simulink model "Performance_analyzer_system_design "  (R2024b)
settings: Solver=VariableStepAuto  StartTime=0.0  StopTime=10.0  RelTol=1e-3
model workspace (MAT data in the model file):
  armature_current = Simulink.Bus
  armature_current.Alignment = -1
  armature_current.PreserveElementDimensions = 0
  armature_current.DataScope = 'Auto'
  line_current = Simulink.Bus
  line_current.Alignment = -1
  line_current.PreserveElementDimensions = 0
  line_current.DataScope = 'Auto'
  speed = Simulink.Bus
  speed.Alignment = -1
  speed.PreserveElementDimensions = 0
  speed.DataScope = 'Auto'
  voltage = Simulink.Bus
  voltage.Alignment = -1
  voltage.PreserveElementDimensions = 0
  voltage.DataScope = 'Auto'

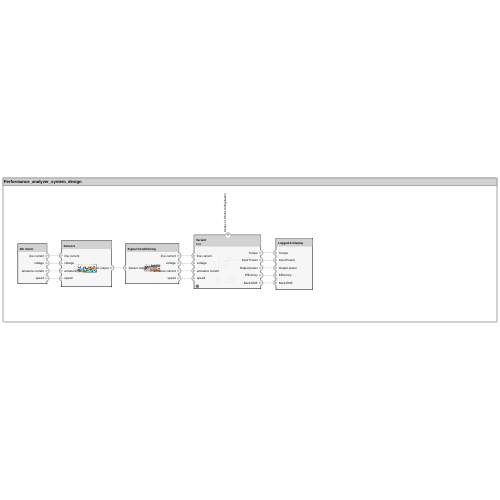
[thumbnail: Simulink's stored preview of the top-level diagram]
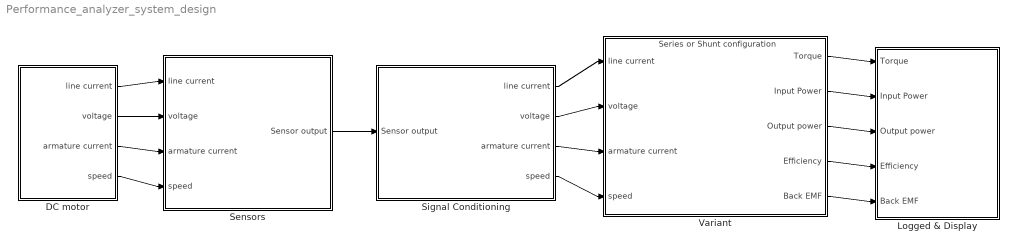
== system Performance_analyzer_system_design  ==
  "DC motor " : Subsystem
      settings: PortSchema="{\"entries\":[{\"content\":{\"headerSize\":26,\"margin\":15,\"minimumSpacing\":25,\"sides\":[{\"content\":{},\"type\":\"ConnectorPlacement.EquallySpacedRectSide\",\"uuid\":\"fe77f070-63ce-405b-ab97-62bbe056a185\"},{\"content\":{\"connectorIds\":[\"Out1\",\"Out2\",\"Out3\",\"Out4\"],\"side\":\"RIGHT\"},\"type\":\"ConnectorPlacement.EquallySpacedRectSide\",\"uuid\":\"342bb455-6b0a-4e5a-b866-05066d23c94e\"},{\"content\":{\"side\":\"TOP\"},\"type\":\"ConnectorPlacement.EquallySpacedRectSide\",\"uuid\":\"f5606689-d5e5-4d9b-a00c-e5773c57f91e\"}]},\"type\":\"ConnectorPlacement.EquallySpacedConnector\",\"uuid\":\"96da3435-ef87-4569-ba54-24bba7686016\"}],\"packageUris\":[\"http://schema.mathworks.com/mf0/Connector/R2020b\"],\"version\":\"1.0\"}"
  Logged & Display : Subsystem
      settings: PortSchema="{\"entries\":[{\"content\":{\"headerSize\":26,\"margin\":15,\"minimumSpacing\":25,\"sides\":[{\"content\":{\"connectorIds\":[\"In1\",\"In2\",\"In3\",\"In4\",\"In5\"]},\"type\":\"ConnectorPlacement.EquallySpacedRectSide\",\"uuid\":\"fe77f070-63ce-405b-ab97-62bbe056a185\"},{\"content\":{\"side\":\"RIGHT\"},\"type\":\"ConnectorPlacement.EquallySpacedRectSide\",\"uuid\":\"342bb455-6b0a-4e5a-b866-05066d23c94e\"},{\"content\":{\"side\":\"TOP\"},\"type\":\"ConnectorPlacement.EquallySpacedRectSide\",\"uuid\":\"f5606689-d5e5-4d9b-a00c-e5773c57f91e\"}]},\"type\":\"ConnectorPlacement.EquallySpacedConnector\",\"uuid\":\"96da3435-ef87-4569-ba54-24bba7686016\"}],\"packageUris\":[\"http://schema.mathworks.com/mf0/Connector/R2020b\"],\"version\":\"1.0\"}"
  Sensors : Subsystem
      settings: PortSchema="{\"entries\":[{\"content\":{\"headerSize\":26,\"margin\":15,\"minimumSpacing\":25,\"sides\":[{\"content\":{\"connectorIds\":[\"In1\",\"In2\",\"In4\",\"In3\"]},\"type\":\"ConnectorPlacement.EquallySpacedRectSide\",\"uuid\":\"fe77f070-63ce-405b-ab97-62bbe056a185\"},{\"content\":{\"connectorIds\":[\"Out1\"],\"side\":\"RIGHT\"},\"type\":\"ConnectorPlacement.EquallySpacedRectSide\",\"uuid\":\"342bb455-6b0a-4e5a-b866-05066d23c94e\"},{\"content\":{\"side\":\"TOP\"},\"type\":\"ConnectorPlacement.EquallySpacedRectSide\",\"uuid\":\"f5606689-d5e5-4d9b-a00c-e5773c57f91e\"}]},\"type\":\"ConnectorPlacement.EquallySpacedConnector\",\"uuid\":\"96da3435-ef87-4569-ba54-24bba7686016\"}],\"packageUris\":[\"http://schema.mathworks.com/mf0/Connector/R2020b\"],\"version\":\"1.0\"}"
  Signal Conditioning : Subsystem
      settings: PortSchema="{\"entries\":[{\"content\":{\"headerSize\":26,\"margin\":15,\"minimumSpacing\":25,\"sides\":[{\"content\":{\"connectorIds\":[\"In1\"]},\"type\":\"ConnectorPlacement.EquallySpacedRectSide\",\"uuid\":\"fe77f070-63ce-405b-ab97-62bbe056a185\"},{\"content\":{\"connectorIds\":[\"Out1\",\"Out2\",\"Out3\",\"Out4\"],\"side\":\"RIGHT\"},\"type\":\"ConnectorPlacement.EquallySpacedRectSide\",\"uuid\":\"342bb455-6b0a-4e5a-b866-05066d23c94e\"},{\"content\":{\"side\":\"TOP\"},\"type\":\"ConnectorPlacement.EquallySpacedRectSide\",\"uuid\":\"f5606689-d5e5-4d9b-a00c-e5773c57f91e\"}]},\"type\":\"ConnectorPlacement.EquallySpacedConnector\",\"uuid\":\"96da3435-ef87-4569-ba54-24bba7686016\"}],\"packageUris\":[\"http://schema.mathworks.com/mf0/Connector/R2020b\"],\"version\":\"1.0\"}"
  "Variant " : Subsystem  Variant=on  VariantControlMode=label  LabelModeActiveChoice=true
      settings: PortSchema="{\"entries\":[{\"content\":{\"headerSize\":38,\"margin\":15,\"minimumSpacing\":25,\"sides\":[{\"content\":{\"connectorIds\":[\"In5\",\"In2\",\"In3\",\"In4\"]},\"type\":\"ConnectorPlacement.EquallySpacedRectSide\",\"uuid\":\"36cdd916-c149-4387-8939-ceee2045fdbf\"},{\"content\":{\"connectorIds\":[\"Out1\",\"Out2\",\"Out3\",\"Out4\",\"Out5\"],\"side\":\"RIGHT\"},\"type\":\"ConnectorPlacement.EquallySpacedRectSide\",\"uuid\":\"dfba5cb6-32b9-4a60-99fd-dc51f99e4758\"},{\"content\":{\"connectorIds\":[\"In1\"],\"side\":\"TOP\"},\"type\":\"ConnectorPlacement.EquallySpacedRectSide\",\"uuid\":\"132443df-21ca-4ae9-8a0a-b36c74e58402\"}]},\"type\":\"ConnectorPlacement.EquallySpacedConnector\",\"uuid\":\"d418145c-b64b-4ad8-a2bf-d585747e29fa\"}],\"packageUris\":[\"http://schema.mathworks.com/mf0/Connector/R2020b\"],\"version\":\"1.0\"}"
  wires:
  "DC motor "/2(voltage) -> Sensors/2(voltage)
  "DC motor "/1(line current) -> Sensors/1(line current)
  "DC motor "/3(armature current) -> Sensors/4(armature current)
  "DC motor "/4(speed) -> Sensors/3(speed)
  Sensors/1(Sensor output) -> Signal Conditioning/1(Sensor output)
  "Variant "/3(Output power) -> Logged & Display/3(Output power)
  "Variant "/1(Torque) -> Logged & Display/1(Torque)
  "Variant "/2(Input Power) -> Logged & Display/2(Input Power)
  "Variant "/4(Efficiency) -> Logged & Display/4(Efficiency)
  "Variant "/5(Back EMF) -> Logged & Display/5(Back EMF)
  Signal Conditioning/1(line current) -> "Variant "/5(line current)
  Signal Conditioning/2(voltage) -> "Variant "/2(voltage)
  Signal Conditioning/3(armature current) -> "Variant "/3(armature current)
  Signal Conditioning/4(speed) -> "Variant "/4(speed)

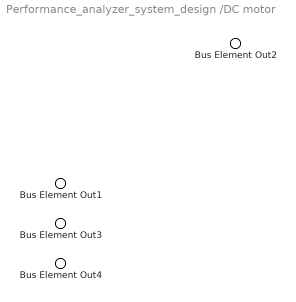
== system Performance_analyzer_system_design /DC motor  ==
  Bus Element Out2 : Outport  PortName=line current
  Bus Element Out1 : Outport  Port=2  PortName=voltage
  Bus Element Out3 : Outport  Port=3  PortName=armature current
  Bus Element Out4 : Outport  Port=4  PortName=speed

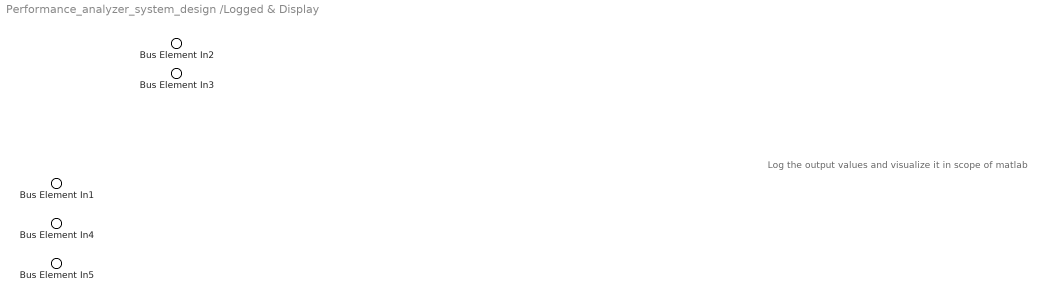
== system Performance_analyzer_system_design /Logged & Display ==
  note: Log the output values and visualize it in scope of matlab
  Bus Element In2 : Inport  PortName=Torque
  Bus Element In3 : Inport  Port=2  PortName=Input Power
  Bus Element In1 : Inport  Port=3  PortName=Output power
  Bus Element In4 : Inport  Port=4  PortName=Efficiency
  Bus Element In5 : Inport  Port=5  PortName=Back EMF

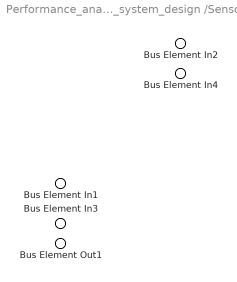
== system Performance_analyzer_system_design /Sensors ==
  Bus Element In2 : Inport  PortName=line current
  Bus Element In1 : Inport  Port=2  PortName=voltage
  Bus Element In4 : Inport  Port=3  PortName=speed
  Bus Element In3 : Inport  Port=4  PortName=armature current
  Bus Element Out1 : Outport  PortName=Sensor output

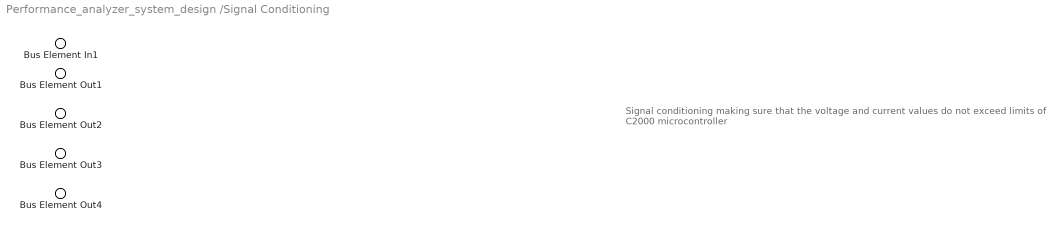
== system Performance_analyzer_system_design /Signal Conditioning ==
  note: Signal conditioning making sure that the voltage and current values do not exceed limits of C2000 microcontroller
  Bus Element In1 : Inport  PortName=Sensor output
  Bus Element Out1 : Outport  PortName=line current
  Bus Element Out2 : Outport  Port=2  PortName=voltage
  Bus Element Out3 : Outport  Port=3  PortName=armature current
  Bus Element Out4 : Outport  Port=4  PortName=speed

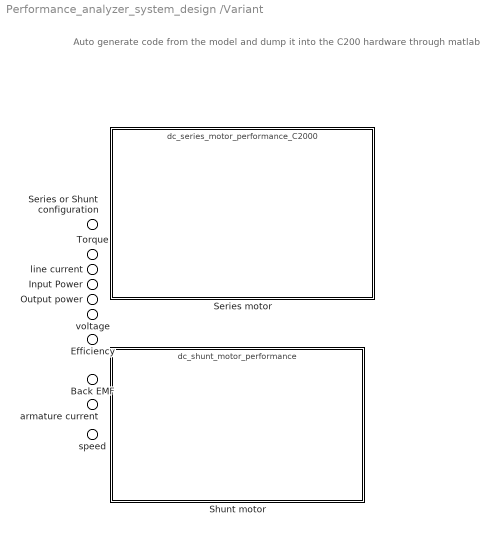
== system Performance_analyzer_system_design /Variant  ==
  note: Auto generate code from the model and dump it into the C200 hardware through matlab
  Series or Shunt configuration : Inport
  voltage : Inport  Port=2
  armature current : Inport  Port=3
  speed : Inport  Port=4
  line current : Inport  Port=5
  Series motor : ModelReference  ModelNameDialog=dc_series_motor_performance_C2000  SimulationMode=Normal  VariantControl=true  ModelReferenceVersion=16.4
      settings: PortSchema="{\"entries\":[{\"content\":{\"headerSize\":38,\"margin\":15,\"minimumSpacing\":25,\"sides\":[{\"content\":{},\"type\":\"ConnectorPlacement.EquallySpacedRectSide\",\"uuid\":\"ca515371-78ed-43aa-8c5e-59f5260d7752\"},{\"content\":{\"side\":\"RIGHT\"},\"type\":\"ConnectorPlacement.EquallySpacedRectSide\",\"uuid\":\"0b87167e-7cb1-4516-8310-f941e0e89f1d\"},{\"content\":{\"side\":\"TOP\"},\"type\":\"ConnectorPlacement.EquallySpacedRectSide\",\"uuid\":\"6bed7271-0f54-4cde-a742-6c69b61fbe37\"}]},\"type\":\"ConnectorPlacement.EquallySpacedConnector\",\"uuid\":\"1715e16b-0077-4a7a-a08a-08f9e25d5dfa\"}],\"packageUris\":[\"http://schema.mathworks.com/mf0/Connector/R2020b\"],\"version\":\"1.0\"}"
  Shunt motor : ModelReference  ModelNameDialog=dc_shunt_motor_performance  SimulationMode=Normal  VariantControl=false  ModelReferenceVersion=16.13
      settings: PortSchema="{\"entries\":[{\"content\":{\"headerSize\":38,\"margin\":15,\"minimumSpacing\":25,\"sides\":[{\"content\":{},\"type\":\"ConnectorPlacement.EquallySpacedRectSide\",\"uuid\":\"ca515371-78ed-43aa-8c5e-59f5260d7752\"},{\"content\":{\"side\":\"RIGHT\"},\"type\":\"ConnectorPlacement.EquallySpacedRectSide\",\"uuid\":\"0b87167e-7cb1-4516-8310-f941e0e89f1d\"},{\"content\":{\"side\":\"TOP\"},\"type\":\"ConnectorPlacement.EquallySpacedRectSide\",\"uuid\":\"6bed7271-0f54-4cde-a742-6c69b61fbe37\"}]},\"type\":\"ConnectorPlacement.EquallySpacedConnector\",\"uuid\":\"1715e16b-0077-4a7a-a08a-08f9e25d5dfa\"}],\"packageUris\":[\"http://schema.mathworks.com/mf0/Connector/R2020b\"],\"version\":\"1.0\"}"
  Torque : Outport
  Input Power : Outport  Port=2
  Output power : Outport  Port=3
  Efficiency : Outport  Port=4
  Back EMF : Outport  Port=5
